simulink model "Lab2_simulink_plc"  (R2023b)
settings: Solver=FixedStepAuto  StartTime=0.0  StopTime=inf  RelTol=1e-3
InitFcn:
  | d13_init.PRE = 50;
undefined symbols (not defined in the file or in its folder): BOOL, DINT, OTL, slplc
libraries: arduinolib x9
(part 5 of 31)

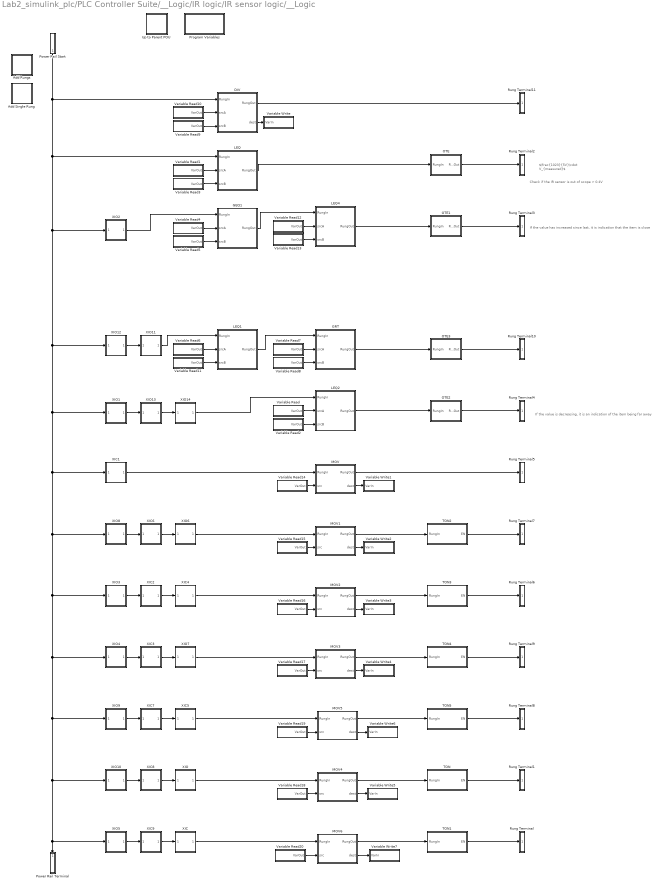
== system Lab2_simulink_plc/PLC Controller Suite/__Logic/IR logic/IR sensor logic/__Logic ==
  note: If the value is decreasing, it is an indication of the item being far away
  note: If the value has increased since last, it is indication that the item is close
  note: $\frac{1023}{5V}\cdot V_{measured}$
  note: Check if the IR sensor is out of scope > 0.4V
  Add Rungs : Subsystem  DisableCoverage=on  TreatAsAtomicUnit=on  mask{PLCRungNumberToAdd=2, PLCBlockType=AddRungs}
      mask prompts: PLCRungNumberToAdd "Rung Number:"
      mask Help: web([docroot '/plccoder/ref/addrungs' '.html']);
      mask DialogControl PLCAddRungs Prompt: Add Rungs
      mask DialogControl PLCRungAddButton Prompt: Add
      mask DialogControl PLCRungAddButton Callback: slplc.callbacks.addLadderRungs(gcb);
      mask Display: disp(sprintf(['Add' newline 'Rungs']));
  Add Single Rung : Subsystem  DisableCoverage=on  TreatAsAtomicUnit=on  mask{PLCRungNumberToAdd=1, PLCBlockType=AddSingleRung}
      settings: OpenFcn=slplc.callbacks.addLadderRungs(gcb);
      mask Help: web([docroot '/plccoder/ref/addsinglerung' '.html']);
      mask DialogControl PLCAddRung Prompt: Add Single Rung
      mask Display: disp(sprintf(['Add' newline '1 Rung']));
  DIV : Subsystem  Priority=4  TreatAsAtomicUnit=on  mask{PLCBlockType=DIV, PLCPOUType=stdFC}
      mask Help: web([docroot '/plccoder/ref/div' '.html']);
      mask DialogControl PLCBlockTitle Prompt: Divide (DIV)
      mask DialogControl PLCBlockDesc Prompt: Divide Source A by Source B.
      mask Display: disp('DIV');
  GEQ1 : Subsystem  Priority=15  TreatAsAtomicUnit=on  mask{PLCBlockType=GEQ, PLCPOUType=stdFC}
      mask Help: web([docroot '/plccoder/ref/geq' '.html']);
      mask DialogControl PLCBlockTitle Prompt: Greater Than or Equal To (GEQ)
      mask DialogControl PLCBlockDesc Prompt: Test whether Source A is greater than or equal to Source B.
      mask Display: disp('GEQ');
  GRT : Subsystem  Priority=28  TreatAsAtomicUnit=on  mask{PLCBlockType=GRT, PLCPOUType=stdFC}
      mask Help: web([docroot '/plccoder/ref/grt' '.html']);
      mask DialogControl PLCBlockTitle Prompt: Greater Than (GRT)
      mask DialogControl PLCBlockDesc Prompt: Test whether Source A is greater than Source B.
      mask Display: disp('GRT');
  LEQ : Subsystem  Priority=9  TreatAsAtomicUnit=on  mask{PLCBlockType=LEQ, PLCPOUType=stdFC}
      mask Help: web([docroot '/plccoder/ref/leq' '.html']);
      mask DialogControl PLCBlockTitle Prompt: Less Than or Equal To (LEQ)
      mask DialogControl PLCBlockDesc Prompt: Test whether Source A is less than or equal to Source B.
      mask Display: disp('LEQ');
  LEQ1 : Subsystem  Priority=25  TreatAsAtomicUnit=on  mask{PLCBlockType=LEQ, PLCPOUType=stdFC}
      mask Help: web([docroot '/plccoder/ref/leq' '.html']);
      mask DialogControl PLCBlockTitle Prompt: Less Than or Equal To (LEQ)
      mask DialogControl PLCBlockDesc Prompt: Test whether Source A is less than or equal to Source B.
      mask Display: disp('LEQ');
  LEQ2 : Subsystem  Priority=36  TreatAsAtomicUnit=on  mask{PLCBlockType=LEQ, PLCPOUType=stdFC}
      mask Help: web([docroot '/plccoder/ref/leq' '.html']);
      mask DialogControl PLCBlockTitle Prompt: Less Than or Equal To (LEQ)
      mask DialogControl PLCBlockDesc Prompt: Test whether Source A is less than or equal to Source B.
      mask Display: disp('LEQ');
  LEQ4 : Subsystem  Priority=18  TreatAsAtomicUnit=on  mask{PLCBlockType=LEQ, PLCPOUType=stdFC}
      mask Help: web([docroot '/plccoder/ref/leq' '.html']);
      mask DialogControl PLCBlockTitle Prompt: Less Than or Equal To (LEQ)
      mask DialogControl PLCBlockDesc Prompt: Test whether Source A is less than or equal to Source B.
      mask Display: disp('LEQ');
  MOV : Subsystem  Priority=41  TreatAsAtomicUnit=on  mask{PLCBlockType=MOV, PLCPOUType=stdFC}
      mask Help: web([docroot '/plccoder/ref/mov' '.html']);
      mask DialogControl PLCBlockTitle Prompt: Move (MOV)
      mask DialogControl PLCBlockDesc Prompt: Move a copy of the Source to the Destination. The Source remains unchanged.
      mask Display: disp('MOV');
  MOV1 : Subsystem  Priority=48  TreatAsAtomicUnit=on  mask{PLCBlockType=MOV, PLCPOUType=stdFC}
      mask Help: web([docroot '/plccoder/ref/mov' '.html']);
      mask DialogControl PLCBlockTitle Prompt: Move (MOV)
      mask DialogControl PLCBlockDesc Prompt: Move a copy of the Source to the Destination. The Source remains unchanged.
      mask Display: disp('MOV');
  MOV2 : Subsystem  Priority=56  TreatAsAtomicUnit=on  mask{PLCBlockType=MOV, PLCPOUType=stdFC}
      mask Help: web([docroot '/plccoder/ref/mov' '.html']);
      mask DialogControl PLCBlockTitle Prompt: Move (MOV)
      mask DialogControl PLCBlockDesc Prompt: Move a copy of the Source to the Destination. The Source remains unchanged.
      mask Display: disp('MOV');
  MOV3 : Subsystem  Priority=64  TreatAsAtomicUnit=on  mask{PLCBlockType=MOV, PLCPOUType=stdFC}
      mask Help: web([docroot '/plccoder/ref/mov' '.html']);
      mask DialogControl PLCBlockTitle Prompt: Move (MOV)
      mask DialogControl PLCBlockDesc Prompt: Move a copy of the Source to the Destination. The Source remains unchanged.
      mask Display: disp('MOV');
  MOV4 : Subsystem  Priority=80  TreatAsAtomicUnit=on  mask{PLCBlockType=MOV, PLCPOUType=stdFC}
      mask Help: web([docroot '/plccoder/ref/mov' '.html']);
      mask DialogControl PLCBlockTitle Prompt: Move (MOV)
      mask DialogControl PLCBlockDesc Prompt: Move a copy of the Source to the Destination. The Source remains unchanged.
      mask Display: disp('MOV');
  MOV5 : Subsystem  Priority=72  TreatAsAtomicUnit=on  mask{PLCBlockType=MOV, PLCPOUType=stdFC}
      mask Help: web([docroot '/plccoder/ref/mov' '.html']);
      mask DialogControl PLCBlockTitle Prompt: Move (MOV)
      mask DialogControl PLCBlockDesc Prompt: Move a copy of the Source to the Destination. The Source remains unchanged.
      mask Display: disp('MOV');
  MOV6 : Subsystem  Priority=88  TreatAsAtomicUnit=on  mask{PLCBlockType=MOV, PLCPOUType=stdFC}
      mask Help: web([docroot '/plccoder/ref/mov' '.html']);
      mask DialogControl PLCBlockTitle Prompt: Move (MOV)
      mask DialogControl PLCBlockDesc Prompt: Move a copy of the Source to the Destination. The Source remains unchanged.
      mask Display: disp('MOV');
  OTE : Subsystem  Priority=10  TreatAsAtomicUnit=on  mask{PLCOperandTag=out_of_scope, PLCBlockType=OTE, PLCPOUType=Coil, PLCOperandExpression=xxx_PLC_VAR_out_of_scope}
      mask prompts: PLCOperandTag "Operand Tag:"
      mask init:
      | slplc.callbacks.initInstruction(gcb);
      settings: AttributesFormatString=%<PLCOperandTag>
      mask Help: web([docroot '/plccoder/ref/ote' '.html']);
      mask DialogControl PLCBlockTitle Prompt: Output Energize (OTE)
  OTE1 : Subsystem  Priority=19  TreatAsAtomicUnit=on  mask{PLCOperandTag=close, PLCBlockType=OTE, PLCPOUType=Coil, PLCOperandExpression=xxx_PLC_VAR_close}
      mask prompts: PLCOperandTag "Operand Tag:"
      mask init:
      | slplc.callbacks.initInstruction(gcb);
      settings: AttributesFormatString=%<PLCOperandTag>
      mask Help: web([docroot '/plccoder/ref/ote' '.html']);
      mask DialogControl PLCBlockTitle Prompt: Output Energize (OTE)
  OTE2 : Subsystem  Priority=37  TreatAsAtomicUnit=on  mask{PLCOperandTag=far, PLCBlockType=OTE, PLCPOUType=Coil, PLCOperandExpression=xxx_PLC_VAR_far}
      mask prompts: PLCOperandTag "Operand Tag:"
      mask init:
      | slplc.callbacks.initInstruction(gcb);
      settings: AttributesFormatString=%<PLCOperandTag>
      mask Help: web([docroot '/plccoder/ref/ote' '.html']);
      mask DialogControl PLCBlockTitle Prompt: Output Energize (OTE)
  OTE3 : Subsystem  Priority=29  TreatAsAtomicUnit=on  mask{PLCOperandTag=half, PLCBlockType=OTE, PLCPOUType=Coil, PLCOperandExpression=xxx_PLC_VAR_half}
      mask prompts: PLCOperandTag "Operand Tag:"
      mask init:
      | slplc.callbacks.initInstruction(gcb);
      settings: AttributesFormatString=%<PLCOperandTag>
      mask Help: web([docroot '/plccoder/ref/ote' '.html']);
      mask DialogControl PLCBlockTitle Prompt: Output Energize (OTE)
  Power Rail Start : Subsystem  Priority=1  DisableCoverage=on  TreatAsAtomicUnit=on  mask{PLCBlockType=PowerRailStart}
      mask Help: web([docroot '/plccoder/ref/powerrailstart' '.html']);
      mask DialogControl PLCBlockTitle Prompt: Power Rail Start
      mask DialogControl PLCBlockDesc Prompt: This is the ladder diagram left power rail start block.
  Power Rail Terminal : Subsystem  DisableCoverage=on  TreatAsAtomicUnit=on  mask{PLCBlockType=PowerRailTerminal}
      mask Help: web([docroot '/plccoder/ref/powerrailterminal' '.html']);
      mask DialogControl PLCBlockTitle Prompt: Power Rail Terminal
      mask DialogControl PLCBlockDesc Prompt: This is the ladder diagram left power rail terminal block.
  Program Variables : Subsystem  Tag=ProgramVariableSS  DisableCoverage=on  TreatAsAtomicUnit=on  mask{PLCVarclose_constantName=close_constant, PLCVarclose_constantScope=Local, PLCVarclose_constantPortType=Hidden, PLCVarclose_constantPortIndex=21, PLCVarclose_constantDataType=uint16, PLCVarclose_constantSize=1, PLCVarclose_constantInitialValue=800, PLCVarclose_constantToDelete=off, PLCVarconstantName=constant, PLCVarconstantScope=Local, PLCVarconstantPortType=Hidden, PLCVarconstantPortIndex=21, PLCVarconstantDataType=uint16, PLCVarconstantSize=1, PLCVarconstantInitialValue=3, PLCVarconstantToDelete=off, PLCVarduty_cycleName=duty_cycle, PLCVarduty_cycleScope=Local, PLCVarduty_cyclePortType=Hidden, PLCVarduty_cyclePortIndex=21, PLCVarduty_cycleDataType=uint8, PLCVarduty_cycleSize=1, PLCVarduty_cycleInitialValue=0, PLCVarduty_cycleToDelete=off, PLCVarfarName=far, PLCVarfarScope=Local, PLCVarfarPortType=Hidden, PLCVarfarPortIndex=21, PLCVarfarDataType=BOOL, PLCVarfarSize=1, PLCVarfarInitialValue=false, PLCVarfarToDelete=off, PLCVarfar_constantName=far_constant, PLCVarfar_constantScope=Local, PLCVarfar_constantPortType=Hidden, PLCVarfar_constantPortIndex=21, PLCVarfar_constantDataType=uint16, PLCVarfar_constantSize=1, PLCVarfar_constantInitialValue=123, PLCVarfar_constantToDelete=off, PLCVarfast_offName=fast_off, PLCVarfast_offScope=Local, PLCVarfast_offPortType=Hidden, PLCVarfast_offPortIndex=21, PLCVarfast_offDataType=Bus: TIMER, PLCVarfast_offSize=1, PLCVarfast_offInitialValue=green_fast, PLCVarfast_offToDelete=off, PLCVarfast_onName=fast_on, PLCVarfast_onScope=Local, PLCVarfast_onPortType=Hidden, PLCVarfast_onPortIndex=21, PLCVarfast_onDataType=Bus: TIMER, PLCVarfast_onSize=1, PLCVarfast_onInitialValue=green_fast, PLCVarfast_onToDelete=off, PLCVargreen_offName=green_off, PLCVargreen_offScope=Local, PLCVargreen_offPortType=Hidden, PLCVargreen_offPortIndex=21, PLCVargreen_offDataType=uint8, PLCVargreen_offSize=1, PLCVargreen_offInitialValue=0, PLCVargreen_offToDelete=off, PLCVarhalfName=half, PLCVarhalfScope=Local, PLCVarhalfPortType=Hidden, PLCVarhalfPortIndex=21, PLCVarhalfDataType=boolean, PLCVarhalfSize=1, PLCVarhalfInitialValue=false, PLCVarhalfToDelete=off, PLCVarhalf_constantName=half_constant, PLCVarhalf_constantScope=Local, PLCVarhalf_constantPortType=Hidden, PLCVarhalf_constantPortIndex=21, PLCVarhalf_constantDataType=DINT, PLCVarhalf_constantSize=1, PLCVarhalf_constantInitialValue=245, PLCVarhalf_constantToDelete=off, PLCVarmedium_offName=medium_off, PLCVarmedium_offScope=Local, PLCVarmedium_offPortType=Hidden, PLCVarmedium_offPortIndex=21, PLCVarmedium_offDataType=Bus: TIMER, PLCVarmedium_offSize=1, PLCVarmedium_offInitialValue=green_medium, PLCVarmedium_offToDelete=off, PLCVarmedium_onName=medium_on, PLCVarmedium_onScope=Local, PLCVarmedium_onPortType=Hidden, PLCVarmedium_onPortIndex=21, PLCVarmedium_onDataType=Bus: TIMER, PLCVarmedium_onSize=1, PLCVarmedium_onInitialValue=green_medium, PLCVarmedium_onToDelete=off, PLCVarout_of_scopeName=out_of_scope, PLCVarout_of_scopeScope=Local, PLCVarout_of_scopePortType=Hidden, PLCVarout_of_scopePortIndex=21, PLCVarout_of_scopeDataType=boolean, PLCVarout_of_scopeSize=1, PLCVarout_of_scopeInitialValue=false, PLCVarout_of_scopeToDelete=off, PLCVarscope_constantName=scope_constant, PLCVarscope_constantScope=Local, PLCVarscope_constantPortType=Hidden, PLCVarscope_constantPortIndex=21, PLCVarscope_constantDataType=uint16, PLCVarscope_constantSize=1, PLCVarscope_constantInitialValue=72, PLCVarscope_constantToDelete=off, PLCVarslow_offName=slow_off, PLCVarslow_offScope=Local, PLCVarslow_offPortType=Hidden, PLCVarslow_offPortIndex=21, PLCVarslow_offDataType=Bus: TIMER, PLCVarslow_offSize=1, PLCVarslow_offInitialValue=green_slow, PLCVarslow_offToDelete=off, PLCVarslow_onName=slow_on, PLCVarslow_onScope=Local, PLCVarslow_onPortType=Hidden, PLCVarslow_onPortIndex=21, PLCVarslow_onDataType=Bus: TIMER, PLCVarslow_onSize=1, PLCVarslow_onInitialValue=green_slow, PLCVarslow_onToDelete=off, PLCVarGreenLedName=GreenLed, PLCVarGreenLedScope=External, PLCVarGreenLedPortType=Hidden, PLCVarGreenLedPortIndex=21, PLCVarGreenLedDataType=DINT, PLCVarGreenLedSize=-1, PLCVarGreenLedInitialValue=0, PLCVarGreenLedToDelete=off, PLCVarIR_value_copyName=IR_value_copy, PLCVarIR_value_copyScope=External, PLCVarIR_value_copyPortType=Hidden, PLCVarIR_value_copyPortIndex=21, PLCVarIR_value_copyDataType=DINT, PLCVarIR_value_copySize=-1, PLCVarIR_value_copyInitialValue=0, PLCVarIR_value_copyToDelete=off, PLCVarcloseName=close, PLCVarcloseScope=External, PLCVarclosePortType=Hidden, PLCVarclosePortIndex=21, PLCVarcloseDataType=DINT, PLCVarcloseSize=-1, PLCVarcloseInitialValue=0, PLCVarcloseToDelete=off, PLCVarrelay_controlName=relay_control, PLCVarrelay_controlScope=External, PLCVarrelay_controlPortType=Hidden, PLCVarrelay_controlPortIndex=21, PLCVarrelay_controlDataType=DINT, PLCVarrelay_controlSize=-1, PLCVarrelay_controlInitialValue=0, PLCVarrelay_controlToDelete=off, PLCVarrelay_valueName=relay_value, PLCVarrelay_valueScope=External, PLCVarrelay_valuePortType=Hidden, PLCVarrelay_valuePortIndex=21, PLCVarrelay_valueDataType=DINT, PLCVarrelay_valueSize=-1, PLCVarrelay_valueInitialValue=0, PLCVarrelay_valueToDelete=off}
      mask init:
      | slplc.callbacks.initPOUVarSS(gcb);
      settings: OpenFcn=slplc.callbacks.openProgramVariableSS(gcb);
      mask Help: web([docroot '/plccoder/ref/programvariables' '.html']);
      mask TypeOptions PLCVarclose_constantScope: Local | External
      mask Callback PLCVarclose_constantScope: slplc.callbacks.updatePOUVarSS(gcb);
      mask TypeOptions PLCVarclose_constantPortType: Hidden | Inport | Outport
      mask Callback PLCVarclose_constantPortType: slplc.callbacks.updatePOUVarSS(gcb);
      mask TypeOptions PLCVarclose_constantPortIndex: 1 | 2 | 3 | 4 | 5 | 6 | 7 | 8 | 9 | 10 | 11 | 12 | 13 | 14 | 15 | 16 | 17 | 18 | 19 | 20 | 21
      mask TypeOptions PLCVarconstantScope: Local | External
      mask Callback PLCVarconstantScope: slplc.callbacks.updatePOUVarSS(gcb);
      mask TypeOptions PLCVarconstantPortType: Hidden | Inport | Outport
      mask Callback PLCVarconstantPortType: slplc.callbacks.updatePOUVarSS(gcb);
      mask TypeOptions PLCVarconstantPortIndex: 1 | 2 | 3 | 4 | 5 | 6 | 7 | 8 | 9 | 10 | 11 | 12 | 13 | 14 | 15 | 16 | 17 | 18 | 19 | 20 | 21
      mask TypeOptions PLCVarduty_cycleScope: Local | External
      mask Callback PLCVarduty_cycleScope: slplc.callbacks.updatePOUVarSS(gcb);
      mask TypeOptions PLCVarduty_cyclePortType: Hidden | Inport | Outport
      mask Callback PLCVarduty_cyclePortType: slplc.callbacks.updatePOUVarSS(gcb);
      mask TypeOptions PLCVarduty_cyclePortIndex: 1 | 2 | 3 | 4 | 5 | 6 | 7 | 8 | 9 | 10 | 11 | 12 | 13 | 14 | 15 | 16 | 17 | 18 | 19 | 20 | 21
      mask TypeOptions PLCVarfarScope: Local | External
      mask Callback PLCVarfarScope: slplc.callbacks.updatePOUVarSS(gcb);
      mask TypeOptions PLCVarfarPortType: Hidden | Inport | Outport
      mask Callback PLCVarfarPortType: slplc.callbacks.updatePOUVarSS(gcb);
      mask TypeOptions PLCVarfarPortIndex: 1 | 2 | 3 | 4 | 5 | 6 | 7 | 8 | 9 | 10 | 11 | 12 | 13 | 14 | 15 | 16 | 17 | 18 | 19 | 20 | 21
      mask TypeOptions PLCVarfar_constantScope: Local | External
      mask Callback PLCVarfar_constantScope: slplc.callbacks.updatePOUVarSS(gcb);
      mask TypeOptions PLCVarfar_constantPortType: Hidden | Inport | Outport
      mask Callback PLCVarfar_constantPortType: slplc.callbacks.updatePOUVarSS(gcb);
      mask TypeOptions PLCVarfar_constantPortIndex: 1 | 2 | 3 | 4 | 5 | 6 | 7 | 8 | 9 | 10 | 11 | 12 | 13 | 14 | 15 | 16 | 17 | 18 | 19 | 20 | 21
      mask TypeOptions PLCVarfast_offScope: Local | External
      mask Callback PLCVarfast_offScope: slplc.callbacks.updatePOUVarSS(gcb);
      mask TypeOptions PLCVarfast_offPortType: Hidden | Inport | Outport
      mask Callback PLCVarfast_offPortType: slplc.callbacks.updatePOUVarSS(gcb);
      mask TypeOptions PLCVarfast_offPortIndex: 1 | 2 | 3 | 4 | 5 | 6 | 7 | 8 | 9 | 10 | 11 | 12 | 13 | 14 | 15 | 16 | 17 | 18 | 19 | 20 | 21
      mask TypeOptions PLCVarfast_onScope: Local | External
      mask Callback PLCVarfast_onScope: slplc.callbacks.updatePOUVarSS(gcb);
      mask TypeOptions PLCVarfast_onPortType: Hidden | Inport | Outport
      mask Callback PLCVarfast_onPortType: slplc.callbacks.updatePOUVarSS(gcb);
      mask TypeOptions PLCVarfast_onPortIndex: 1 | 2 | 3 | 4 | 5 | 6 | 7 | 8 | 9 | 10 | 11 | 12 | 13 | 14 | 15 | 16 | 17 | 18 | 19 | 20 | 21
      mask TypeOptions PLCVargreen_offScope: Local | External
      mask Callback PLCVargreen_offScope: slplc.callbacks.updatePOUVarSS(gcb);
      mask TypeOptions PLCVargreen_offPortType: Hidden | Inport | Outport
      mask Callback PLCVargreen_offPortType: slplc.callbacks.updatePOUVarSS(gcb);
      mask TypeOptions PLCVargreen_offPortIndex: 1 | 2 | 3 | 4 | 5 | 6 | 7 | 8 | 9 | 10 | 11 | 12 | 13 | 14 | 15 | 16 | 17 | 18 | 19 | 20 | 21
      mask TypeOptions PLCVarhalfScope: Local | External
      mask Callback PLCVarhalfScope: slplc.callbacks.updatePOUVarSS(gcb);
      mask TypeOptions PLCVarhalfPortType: Hidden | Inport | Outport
      mask Callback PLCVarhalfPortType: slplc.callbacks.updatePOUVarSS(gcb);
      mask TypeOptions PLCVarhalfPortIndex: 1 | 2 | 3 | 4 | 5 | 6 | 7 | 8 | 9 | 10 | 11 | 12 | 13 | 14 | 15 | 16 | 17 | 18 | 19 | 20 | 21
      mask TypeOptions PLCVarhalf_constantScope: Local | External
      mask Callback PLCVarhalf_constantScope: slplc.callbacks.updatePOUVarSS(gcb);
      mask TypeOptions PLCVarhalf_constantPortType: Hidden | Inport | Outport
      mask Callback PLCVarhalf_constantPortType: slplc.callbacks.updatePOUVarSS(gcb);
      mask TypeOptions PLCVarhalf_constantPortIndex: 1 | 2 | 3 | 4 | 5 | 6 | 7 | 8 | 9 | 10 | 11 | 12 | 13 | 14 | 15 | 16 | 17 | 18 | 19 | 20 | 21
      mask TypeOptions PLCVarmedium_offScope: Local | External
      mask Callback PLCVarmedium_offScope: slplc.callbacks.updatePOUVarSS(gcb);
      mask TypeOptions PLCVarmedium_offPortType: Hidden | Inport | Outport
      mask Callback PLCVarmedium_offPortType: slplc.callbacks.updatePOUVarSS(gcb);
      mask TypeOptions PLCVarmedium_offPortIndex: 1 | 2 | 3 | 4 | 5 | 6 | 7 | 8 | 9 | 10 | 11 | 12 | 13 | 14 | 15 | 16 | 17 | 18 | 19 | 20 | 21
      mask TypeOptions PLCVarmedium_onScope: Local | External
      mask Callback PLCVarmedium_onScope: slplc.callbacks.updatePOUVarSS(gcb);
      mask TypeOptions PLCVarmedium_onPortType: Hidden | Inport | Outport
      mask Callback PLCVarmedium_onPortType: slplc.callbacks.updatePOUVarSS(gcb);
      mask TypeOptions PLCVarmedium_onPortIndex: 1 | 2 | 3 | 4 | 5 | 6 | 7 | 8 | 9 | 10 | 11 | 12 | 13 | 14 | 15 | 16 | 17 | 18 | 19 | 20 | 21
      mask TypeOptions PLCVarout_of_scopeScope: Local | External
      mask Callback PLCVarout_of_scopeScope: slplc.callbacks.updatePOUVarSS(gcb);
      mask TypeOptions PLCVarout_of_scopePortType: Hidden | Inport | Outport
      mask Callback PLCVarout_of_scopePortType: slplc.callbacks.updatePOUVarSS(gcb);
      mask TypeOptions PLCVarout_of_scopePortIndex: 1 | 2 | 3 | 4 | 5 | 6 | 7 | 8 | 9 | 10 | 11 | 12 | 13 | 14 | 15 | 16 | 17 | 18 | 19 | 20 | 21
      mask TypeOptions PLCVarscope_constantScope: Local | External
      mask Callback PLCVarscope_constantScope: slplc.callbacks.updatePOUVarSS(gcb);
      mask TypeOptions PLCVarscope_constantPortType: Hidden | Inport | Outport
      mask Callback PLCVarscope_constantPortType: slplc.callbacks.updatePOUVarSS(gcb);
      mask TypeOptions PLCVarscope_constantPortIndex: 1 | 2 | 3 | 4 | 5 | 6 | 7 | 8 | 9 | 10 | 11 | 12 | 13 | 14 | 15 | 16 | 17 | 18 | 19 | 20 | 21
      mask TypeOptions PLCVarslow_offScope: Local | External
      mask Callback PLCVarslow_offScope: slplc.callbacks.updatePOUVarSS(gcb);
      mask TypeOptions PLCVarslow_offPortType: Hidden | Inport | Outport
      mask Callback PLCVarslow_offPortType: slplc.callbacks.updatePOUVarSS(gcb);
      mask TypeOptions PLCVarslow_offPortIndex: 1 | 2 | 3 | 4 | 5 | 6 | 7 | 8 | 9 | 10 | 11 | 12 | 13 | 14 | 15 | 16 | 17 | 18 | 19 | 20 | 21
      mask TypeOptions PLCVarslow_onScope: Local | External
      mask Callback PLCVarslow_onScope: slplc.callbacks.updatePOUVarSS(gcb);
      mask TypeOptions PLCVarslow_onPortType: Hidden | Inport | Outport
      mask Callback PLCVarslow_onPortType: slplc.callbacks.updatePOUVarSS(gcb);
      mask TypeOptions PLCVarslow_onPortIndex: 1 | 2 | 3 | 4 | 5 | 6 | 7 | 8 | 9 | 10 | 11 | 12 | 13 | 14 | 15 | 16 | 17 | 18 | 19 | 20 | 21
      mask TypeOptions PLCVarGreenLedScope: Local | External
      mask Callback PLCVarGreenLedScope: slplc.callbacks.updatePOUVarSS(gcb);
      mask TypeOptions PLCVarGreenLedPortType: Hidden | Inport | Outport
      mask Callback PLCVarGreenLedPortType: slplc.callbacks.updatePOUVarSS(gcb);
      mask TypeOptions PLCVarGreenLedPortIndex: 1 | 2 | 3 | 4 | 5 | 6 | 7 | 8 | 9 | 10 | 11 | 12 | 13 | 14 | 15 | 16 | 17 | 18 | 19 | 20 | 21
      mask TypeOptions PLCVarIR_value_copyScope: Local | External
      mask Callback PLCVarIR_value_copyScope: slplc.callbacks.updatePOUVarSS(gcb);
      mask TypeOptions PLCVarIR_value_copyPortType: Hidden | Inport | Outport
      mask Callback PLCVarIR_value_copyPortType: slplc.callbacks.updatePOUVarSS(gcb);
      mask TypeOptions PLCVarIR_value_copyPortIndex: 1 | 2 | 3 | 4 | 5 | 6 | 7 | 8 | 9 | 10 | 11 | 12 | 13 | 14 | 15 | 16 | 17 | 18 | 19 | 20 | 21
      mask TypeOptions PLCVarcloseScope: Local | External
      mask Callback PLCVarcloseScope: slplc.callbacks.updatePOUVarSS(gcb);
      mask TypeOptions PLCVarclosePortType: Hidden | Inport | Outport
      mask Callback PLCVarclosePortType: slplc.callbacks.updatePOUVarSS(gcb);
      mask TypeOptions PLCVarclosePortIndex: 1 | 2 | 3 | 4 | 5 | 6 | 7 | 8 | 9 | 10 | 11 | 12 | 13 | 14 | 15 | 16 | 17 | 18 | 19 | 20 | 21
      mask TypeOptions PLCVarrelay_controlScope: Local | External
      mask Callback PLCVarrelay_controlScope: slplc.callbacks.updatePOUVarSS(gcb);
      mask TypeOptions PLCVarrelay_controlPortType: Hidden | Inport | Outport
      mask Callback PLCVarrelay_controlPortType: slplc.callbacks.updatePOUVarSS(gcb);
      mask TypeOptions PLCVarrelay_controlPortIndex: 1 | 2 | 3 | 4 | 5 | 6 | 7 | 8 | 9 | 10 | 11 | 12 | 13 | 14 | 15 | 16 | 17 | 18 | 19 | 20 | 21
      mask TypeOptions PLCVarrelay_valueScope: Local | External
      mask Callback PLCVarrelay_valueScope: slplc.callbacks.updatePOUVarSS(gcb);
      mask TypeOptions PLCVarrelay_valuePortType: Hidden | Inport | Outport
      mask Callback PLCVarrelay_valuePortType: slplc.callbacks.updatePOUVarSS(gcb);
      mask TypeOptions PLCVarrelay_valuePortIndex: 1 | 2 | 3 | 4 | 5 | 6 | 7 | 8 | 9 | 10 | 11 | 12 | 13 | 14 | 15 | 16 | 17 | 18 | 19 | 20 | 21
      mask DialogControl PLCBlockTitle Prompt: Program Variable Spreadsheet
      mask DialogControl PLCVarListHeaderName Prompt: Name
      mask DialogControl PLCVarListHeaderScope Prompt: Scope
      mask DialogControl PLCVarListHeaderDataType Prompt: Data Type
      mask DialogControl PLCVarListHeaderSize Prompt: Size
      mask DialogControl PLCVarListHeaderInitialValue Prompt: Initial Value
      mask DialogControl PLCVarListHeaderToDelete Prompt: Delete
  Rung Terminal : Subsystem  Priority=91  DisableCoverage=on  TreatAsAtomicUnit=on  mask{PLCRungTerminalIndex=12, PLCBlockType=RungTerminal}
      settings: AttributesFormatString=Rung %<PLCRungTerminalIndex>
      mask Help: web([docroot '/plccoder/ref/rungterminal' '.html']);
      mask DialogControl PLCBlockTitle Prompt: Rung Terminal
      mask DialogControl PLCBlockDescText Prompt: This is the ladder diagram rung terminal.
  Rung Terminal1 : Subsystem  Priority=83  DisableCoverage=on  TreatAsAtomicUnit=on  mask{PLCRungTerminalIndex=11, PLCBlockType=RungTerminal}
      settings: AttributesFormatString=Rung %<PLCRungTerminalIndex>
      mask Help: web([docroot '/plccoder/ref/rungterminal' '.html']);
      mask DialogControl PLCBlockTitle Prompt: Rung Terminal
      mask DialogControl PLCBlockDescText Prompt: This is the ladder diagram rung terminal.
  Rung Terminal10 : Subsystem  Priority=30  DisableCoverage=on  TreatAsAtomicUnit=on  mask{PLCRungTerminalIndex=4, PLCBlockType=RungTerminal}
      settings: AttributesFormatString=Rung %<PLCRungTerminalIndex>
      mask Help: web([docroot '/plccoder/ref/rungterminal' '.html']);
      mask DialogControl PLCBlockTitle Prompt: Rung Terminal
      mask DialogControl PLCBlockDescText Prompt: This is the ladder diagram rung terminal.
  Rung Terminal11 : Subsystem  Priority=6  DisableCoverage=on  TreatAsAtomicUnit=on  mask{PLCRungTerminalIndex=1, PLCBlockType=RungTerminal}
      settings: AttributesFormatString=Rung %<PLCRungTerminalIndex>
      mask Help: web([docroot '/plccoder/ref/rungterminal' '.html']);
      mask DialogControl PLCBlockTitle Prompt: Rung Terminal
      mask DialogControl PLCBlockDescText Prompt: This is the ladder diagram rung terminal.
  Rung Terminal2 : Subsystem  Priority=11  DisableCoverage=on  TreatAsAtomicUnit=on  mask{PLCRungTerminalIndex=2, PLCBlockType=RungTerminal}
      settings: AttributesFormatString=Rung %<PLCRungTerminalIndex>
      mask Help: web([docroot '/plccoder/ref/rungterminal' '.html']);
      mask DialogControl PLCBlockTitle Prompt: Rung Terminal
      mask DialogControl PLCBlockDescText Prompt: This is the ladder diagram rung terminal.
  Rung Terminal3 : Subsystem  Priority=20  DisableCoverage=on  TreatAsAtomicUnit=on  mask{PLCRungTerminalIndex=3, PLCBlockType=RungTerminal}
      settings: AttributesFormatString=Rung %<PLCRungTerminalIndex>
      mask Help: web([docroot '/plccoder/ref/rungterminal' '.html']);
      mask DialogControl PLCBlockTitle Prompt: Rung Terminal
      mask DialogControl PLCBlockDescText Prompt: This is the ladder diagram rung terminal.
  Rung Terminal4 : Subsystem  Priority=38  DisableCoverage=on  TreatAsAtomicUnit=on  mask{PLCRungTerminalIndex=5, PLCBlockType=RungTerminal}
      settings: AttributesFormatString=Rung %<PLCRungTerminalIndex>
      mask Help: web([docroot '/plccoder/ref/rungterminal' '.html']);
      mask DialogControl PLCBlockTitle Prompt: Rung Terminal
      mask DialogControl PLCBlockDescText Prompt: This is the ladder diagram rung terminal.
  Rung Terminal5 : Subsystem  Priority=43  DisableCoverage=on  TreatAsAtomicUnit=on  mask{PLCRungTerminalIndex=6, PLCBlockType=RungTerminal}
      settings: AttributesFormatString=Rung %<PLCRungTerminalIndex>
      mask Help: web([docroot '/plccoder/ref/rungterminal' '.html']);
      mask DialogControl PLCBlockTitle Prompt: Rung Terminal
      mask DialogControl PLCBlockDescText Prompt: This is the ladder diagram rung terminal.
  Rung Terminal6 : Subsystem  Priority=59  DisableCoverage=on  TreatAsAtomicUnit=on  mask{PLCRungTerminalIndex=8, PLCBlockType=RungTerminal}
      settings: AttributesFormatString=Rung %<PLCRungTerminalIndex>
      mask Help: web([docroot '/plccoder/ref/rungterminal' '.html']);
      mask DialogControl PLCBlockTitle Prompt: Rung Terminal
      mask DialogControl PLCBlockDescText Prompt: This is the ladder diagram rung terminal.
  Rung Terminal7 : Subsystem  Priority=51  DisableCoverage=on  TreatAsAtomicUnit=on  mask{PLCRungTerminalIndex=7, PLCBlockType=RungTerminal}
      settings: AttributesFormatString=Rung %<PLCRungTerminalIndex>
      mask Help: web([docroot '/plccoder/ref/rungterminal' '.html']);
      mask DialogControl PLCBlockTitle Prompt: Rung Terminal
      mask DialogControl PLCBlockDescText Prompt: This is the ladder diagram rung terminal.
  Rung Terminal8 : Subsystem  Priority=75  DisableCoverage=on  TreatAsAtomicUnit=on  mask{PLCRungTerminalIndex=10, PLCBlockType=RungTerminal}
      settings: AttributesFormatString=Rung %<PLCRungTerminalIndex>
      mask Help: web([docroot '/plccoder/ref/rungterminal' '.html']);
      mask DialogControl PLCBlockTitle Prompt: Rung Terminal
      mask DialogControl PLCBlockDescText Prompt: This is the ladder diagram rung terminal.
  Rung Terminal9 : Subsystem  Priority=67  DisableCoverage=on  TreatAsAtomicUnit=on  mask{PLCRungTerminalIndex=9, PLCBlockType=RungTerminal}
      settings: AttributesFormatString=Rung %<PLCRungTerminalIndex>
      mask Help: web([docroot '/plccoder/ref/rungterminal' '.html']);
      mask DialogControl PLCBlockTitle Prompt: Rung Terminal
      mask DialogControl PLCBlockDescText Prompt: This is the ladder diagram rung terminal.
  TON : Subsystem  Priority=82  TreatAsAtomicUnit=on  mask{PLCOperandTag=slow_on, PLCTimerSampleTime=0.2, PLCBlockType=TON, PLCPOUType=stdFB, PLCOperandAccess=ReadWrite, PLCOperandExpression=xxx_PLC_VAR_slow_on}
      mask prompts: PLCOperandTag "Timer Tag:"; PLCTimerSampleTime "Timer Sample Time:"
      mask init:
      | block = gcb;
      | maskObj = Simulink.Mask.get(block);
      | maskObj.Display = sprintf('disp([''%s'' newline newline ''%s'']);', PLCBlockType, PLCOperandTag);
      | slplc.callbacks.initInstruction(block);
      settings: AttributesFormatString=%<PLCBlockType>: %<PLCOperandTag>
      mask Help: web([docroot '/plccoder/ref/ton' '.html']);
      mask DialogControl PLCBlockTitle Prompt: Timer On Delay (TON)
      mask Display: disp(['TON' newline newline 'slow_on']);
  TON1 : Subsystem  Priority=90  TreatAsAtomicUnit=on  mask{PLCOperandTag=slow_off, PLCTimerSampleTime=0.2, PLCBlockType=TON, PLCPOUType=stdFB, PLCOperandAccess=ReadWrite, PLCOperandExpression=xxx_PLC_VAR_slow_off}
      mask prompts: PLCOperandTag "Timer Tag:"; PLCTimerSampleTime "Timer Sample Time:"
      mask init:
      | block = gcb;
      | maskObj = Simulink.Mask.get(block);
      | maskObj.Display = sprintf('disp([''%s'' newline newline ''%s'']);', PLCBlockType, PLCOperandTag);
      | slplc.callbacks.initInstruction(block);
      settings: AttributesFormatString=%<PLCBlockType>: %<PLCOperandTag>
      mask Help: web([docroot '/plccoder/ref/ton' '.html']);
      mask DialogControl PLCBlockTitle Prompt: Timer On Delay (TON)
      mask Display: disp(['TON' newline newline 'slow_off']);
  TON2 : Subsystem  Priority=50  TreatAsAtomicUnit=on  mask{PLCOperandTag=fast_on, PLCTimerSampleTime=0.2, PLCBlockType=TON, PLCPOUType=stdFB, PLCOperandAccess=ReadWrite, PLCOperandExpression=xxx_PLC_VAR_fast_on}
      mask prompts: PLCOperandTag "Timer Tag:"; PLCTimerSampleTime "Timer Sample Time:"
      mask init:
      | block = gcb;
      | maskObj = Simulink.Mask.get(block);
      | maskObj.Display = sprintf('disp([''%s'' newline newline ''%s'']);', PLCBlockType, PLCOperandTag);
      | slplc.callbacks.initInstruction(block);
      settings: AttributesFormatString=%<PLCBlockType>: %<PLCOperandTag>
      mask Help: web([docroot '/plccoder/ref/ton' '.html']);
      mask DialogControl PLCBlockTitle Prompt: Timer On Delay (TON)
      mask Display: disp(['TON' newline newline 'fast_on']);
  TON3 : Subsystem  Priority=58  TreatAsAtomicUnit=on  mask{PLCOperandTag=fast_off, PLCTimerSampleTime=0.2, PLCBlockType=TON, PLCPOUType=stdFB, PLCOperandAccess=ReadWrite, PLCOperandExpression=xxx_PLC_VAR_fast_off}
      mask prompts: PLCOperandTag "Timer Tag:"; PLCTimerSampleTime "Timer Sample Time:"
      mask init:
      | block = gcb;
      | maskObj = Simulink.Mask.get(block);
      | maskObj.Display = sprintf('disp([''%s'' newline newline ''%s'']);', PLCBlockType, PLCOperandTag);
      | slplc.callbacks.initInstruction(block);
      settings: AttributesFormatString=%<PLCBlockType>: %<PLCOperandTag>
      mask Help: web([docroot '/plccoder/ref/ton' '.html']);
      mask DialogControl PLCBlockTitle Prompt: Timer On Delay (TON)
      mask Display: disp(['TON' newline newline 'fast_off']);
  TON4 : Subsystem  Priority=66  TreatAsAtomicUnit=on  mask{PLCOperandTag=medium_on, PLCTimerSampleTime=0.2, PLCBlockType=TON, PLCPOUType=stdFB, PLCOperandAccess=ReadWrite, PLCOperandExpression=xxx_PLC_VAR_medium_on}
      mask prompts: PLCOperandTag "Timer Tag:"; PLCTimerSampleTime "Timer Sample Time:"